simulink model "ofdm_txrx_alamouti"  (Simulink 6.3)
settings: Solver=ode45  StartTime=0.0  StopTime=45000  RelTol=1e-3
InitFcn:
  | ofdm_rx_alamouti_init
StartFcn:
  | ofdm_rx_alamouti_init
values from neighbouring files (symbols this model uses):
  CPLength = 16    [ofdm_tx_alamouti_init.m]
  CRCPolynomial = hex2dec('04c11db7')    [calcTxCRC.m]    (also in ofdm_tx_alamouti_init.m)
  hi = zeros(1,length(cci))    [ofdm_rx_alamouti_init.m]
  hr = zeros(1,length(ccr))    [ofdm_rx_alamouti_init.m]
  max_CP_length = 16    [ofdm_tx_alamouti_init.m]
  max_num_baseRateSymbols = 31    [ofdm_tx_alamouti_init.m]
  max_num_subcarriers = 64    [ofdm_tx_alamouti_init.m]
  max_num_trainingSymbols = 15    [ofdm_tx_alamouti_init.m]
  max_OFDM_symbols = 2047    [ofdm_tx_alamouti_init.m]
  modConstellation_qam16 = 0.75*[1 3 -1 -3]./3    [ofdm_tx_alamouti_init.m]
  modConstellation_qam256 = 0.9375*[3 1 5 7 11 9 13 15 -3 -1 -5 -7 -11 -9 -13 -15]./15    [ofdm_tx_alamouti_init.m]
  modConstellation_qam64 = 0.875*[3 1 5 7 -3 -1 -5 -7]./7    [ofdm_tx_alamouti_init.m]
  modConstellation_qpsk = [1 -1]./sqrt(2)    [ofdm_tx_alamouti_init.m]
  numBaseRateSymbols = 2    [ofdm_tx_alamouti_init.m]
  numFullRateSymbols = numFullRateSymbols + mod(numFullRateSymbols, 2)    [ofdm_tx_alamouti_init.m]
  numSubcarriers = 64    [ofdm_tx_alamouti_init.m]
  numTrainingSymbols = 4    [ofdm_tx_alamouti_init.m]
  pkt_crossCorr_thresh = 0.7    [ofdm_rx_alamouti_init.m]
  pkt_energy_thresh = 0    [ofdm_rx_alamouti_init.m]
  pkt_numPayloadBytes = 24+4+20+20+500    (= 568)    [ofdm_tx_alamouti_init.m]
  pktTiming_count_payloadStart = pktTiming_count_lsStart + pktTiming_ls_num*pktTiming_ls_length    [ofdm_rx_init_packetTimingControl.m]
  preamble = 6*[0 shortsyms_10 longsyms_2]    [ofdm_tx_alamouti_init.m]
  preamble_I = real(preamble)    [ofdm_tx_alamouti_init.m]
  preamble_Q = imag(preamble)    [ofdm_tx_alamouti_init.m]
  RAM_init_size = 4096    [ofdm_tx_alamouti_init.m]
  rx_longCorrCountLoad = 251-16    (= 235)    [ofdm_rx_alamouti_init.m]
  rx_longCorrThresh = 3000    [ofdm_rx_alamouti_init.m]
  RxDataScrambling_Seq = [40 198 78 63 82 173 102 245 48 111 172 115 147 230 216 93 72 65 62 2 205 242 122 90 128 83 105 97 73 10 5 252]    [ofdm_tx_alamouti_init.m]
  simOnly_numSamples = length(preamble)+( (numSubcarriers+CPLength)*(numTrainingSymbols + numBaseRateSymbols + numFullRateSymbols) )    [ofdm_tx_alamouti_init.m]
  train = [train train]    [ofdm_tx_alamouti_init.m]
  TxDataScrambling_Seq = [40 198 78 63 82 173 102 245 48 111 172 115 147 230 216 93 72 65 62 2 205 242 122 90 128 83 105 97 73 10 5 252]    [ofdm_tx_alamouti_init.m]
undefined symbols (not defined in the file or in its folder): CRC_table_gen, gcbh, interpFilter_4, xlBlockLoadCallback
libraries: xbsIndex_r4 x4373, dspmlti4 x4, dspsigops x4, commchan3 x2, simulink x2, commrflib2 x2, commsink2 x2, dspsrcs4 x1
(part 2 of 39)

== system ofdm_txrx_alamouti/BRAM Interface/PLB Pkt Buffer ==
  BRAM_Dout : Inport
  WEn : Inport  Port=2
  Addr : Inport  Port=3
  AddrIn : xbsIndex_r4/Gateway In  arith_type=Unsigned  n_bits=32  bin_pt=0  quantization="Round  (unbiased: +/- Inf)"  overflow=Saturate  period=1  dbl_ovrd=off  timing_constraint=None  locs_specified=off  LOCs={}  block_type=gatewayin
      settings: infoedit="Gateway in block.  Converts inputs of type Simulink integer, double and fixed point to  Xilinx fixed point type.<P><P>Hardware notes:  In hardware these blocks become top level input ports."  block_version=8.2.02
  Byte0 : xbsIndex_r4/Slice  nbits=8  boolean_output=off  mode=Lower Bit Location + Width  bit1=0  base1=MSB of Input  bit0=0  base0=LSB of Input  dbl_ovrd=off  block_type=slice
      settings: infoedit="Extracts a given range of bits from each input sample and presents it at the output.  The output type is ordinarily unsigned with binary point at zero, but can be Boolean when the slice is one bit wide.<P><P>Hardware notes: In hardware this block costs nothing."  block_version=8.2.02
  Byte1 : xbsIndex_r4/Slice  nbits=8  boolean_output=off  mode=Lower Bit Location + Width  bit1=0  base1=MSB of Input  bit0=8  base0=LSB of Input  dbl_ovrd=off  block_type=slice
      settings: infoedit="Extracts a given range of bits from each input sample and presents it at the output.  The output type is ordinarily unsigned with binary point at zero, but can be Boolean when the slice is one bit wide.<P><P>Hardware notes: In hardware this block costs nothing."  block_version=8.2.02
  Byte2 : xbsIndex_r4/Slice  nbits=8  boolean_output=off  mode=Lower Bit Location + Width  bit1=0  base1=MSB of Input  bit0=16  base0=LSB of Input  dbl_ovrd=off  block_type=slice
      settings: infoedit="Extracts a given range of bits from each input sample and presents it at the output.  The output type is ordinarily unsigned with binary point at zero, but can be Boolean when the slice is one bit wide.<P><P>Hardware notes: In hardware this block costs nothing."  block_version=8.2.02
  Byte3 : xbsIndex_r4/Slice  nbits=8  boolean_output=off  mode=Lower Bit Location + Width  bit1=0  base1=MSB of Input  bit0=24  base0=LSB of Input  dbl_ovrd=off  block_type=slice
      settings: infoedit="Extracts a given range of bits from each input sample and presents it at the output.  The output type is ordinarily unsigned with binary point at zero, but can be Boolean when the slice is one bit wide.<P><P>Hardware notes: In hardware this block costs nothing."  block_version=8.2.02
  Byte4 : xbsIndex_r4/Slice  nbits=8  boolean_output=off  mode=Lower Bit Location + Width  bit1=0  base1=MSB of Input  bit0=32  base0=LSB of Input  dbl_ovrd=off  block_type=slice
      settings: infoedit="Extracts a given range of bits from each input sample and presents it at the output.  The output type is ordinarily unsigned with binary point at zero, but can be Boolean when the slice is one bit wide.<P><P>Hardware notes: In hardware this block costs nothing."  block_version=8.2.02
  Byte5 : xbsIndex_r4/Slice  nbits=8  boolean_output=off  mode=Lower Bit Location + Width  bit1=0  base1=MSB of Input  bit0=40  base0=LSB of Input  dbl_ovrd=off  block_type=slice
      settings: infoedit="Extracts a given range of bits from each input sample and presents it at the output.  The output type is ordinarily unsigned with binary point at zero, but can be Boolean when the slice is one bit wide.<P><P>Hardware notes: In hardware this block costs nothing."  block_version=8.2.02
  Byte6 : xbsIndex_r4/Slice  nbits=8  boolean_output=off  mode=Lower Bit Location + Width  bit1=0  base1=MSB of Input  bit0=48  base0=LSB of Input  dbl_ovrd=off  block_type=slice
      settings: infoedit="Extracts a given range of bits from each input sample and presents it at the output.  The output type is ordinarily unsigned with binary point at zero, but can be Boolean when the slice is one bit wide.<P><P>Hardware notes: In hardware this block costs nothing."  block_version=8.2.02
  Byte7 : xbsIndex_r4/Slice  nbits=8  boolean_output=off  mode=Lower Bit Location + Width  bit1=0  base1=MSB of Input  bit0=56  base0=LSB of Input  dbl_ovrd=off  block_type=slice
      settings: infoedit="Extracts a given range of bits from each input sample and presents it at the output.  The output type is ordinarily unsigned with binary point at zero, but can be Boolean when the slice is one bit wide.<P><P>Hardware notes: In hardware this block costs nothing."  block_version=8.2.02
  Byte8 : xbsIndex_r4/Slice  nbits=9  boolean_output=off  mode=Lower Bit Location + Width  bit1=0  base1=MSB of Input  bit0=3  base0=LSB of Input  dbl_ovrd=off  block_type=slice
      settings: infoedit="Extracts a given range of bits from each input sample and presents it at the output.  The output type is ordinarily unsigned with binary point at zero, but can be Boolean when the slice is one bit wide.<P><P>Hardware notes: In hardware this block costs nothing."  block_version=8.2.02
  Concat : xbsIndex_r4/Concat  num_inputs=8  dbl_ovrd=off  block_type=concat
      settings: block_version=8.2.02
  Constant : xbsIndex_r4/Constant  arith_type=Unsigned  const=0  n_bits=1  bin_pt=0  explicit_period=off  period=1  equ=P=C  opselect=C  inp2=PCIN>>17  opr=+  inp1=P  carry=CIN  dbl_ovrd=off  block_type=constant
      settings: block_version=8.2.02
  DataIn : xbsIndex_r4/Gateway In  arith_type=Unsigned  n_bits=64  bin_pt=0  quantization="Round  (unbiased: +/- Inf)"  overflow=Saturate  period=1  dbl_ovrd=off  timing_constraint=None  locs_specified=off  LOCs={}  block_type=gatewayin
      settings: infoedit="Gateway in block.  Converts inputs of type Simulink integer, double and fixed point to  Xilinx fixed point type.<P><P>Hardware notes:  In hardware these blocks become top level input ports."  block_version=8.2.02
  DataOut : xbsIndex_r4/Gateway Out  hdl_port=on  timing_constraint=None  locs_specified=off  LOCs={}  block_type=gatewayout
      settings: infoedit="Gateway out block.  Converts Xilinx fixed point inputs into ouputs of type Simulink integer, double, or fixed point.<P><P>Hardware notes:  In hardware these blocks become top level output ports or are discarded, depending on how they are configured."  block_version=8.2.02
  Delay : xbsIndex_r4/Delay  en=on  latency=1  dbl_ovrd=off  reg_retiming=off  block_type=delay
      settings: infoedit=Hardware notes: A delay line is a chain, each link of which is an SRL16 followed by a flip-flop. If register retiming is enabled, the delay line is a chain of flip-flops.  block_version=8.2.02
  Disregard Subsystem : xbsIndex_r4/Disregard Subsystem  Tag=discardX  block_type=disregard
      settings: block_version=8.2.02
  Gateway Out{,1..3} (4 alike) : xbsIndex_r4/Gateway Out  hdl_port=off  timing_constraint=None  locs_specified=off  LOCs={}  block_type=gatewayout
      settings: infoedit="Gateway out block.  Converts Xilinx fixed point inputs into ouputs of type Simulink integer, double, or fixed point.<P><P>Hardware notes:  In hardware these blocks become top level output ports or are discarded, depending on how they are configured."  block_version=8.2.02
  ROM : xbsIndex_r4/ROM  depth=RAM_init_size/8  initVector=RAM_init_values(8:8:end)  distributed_mem=Block RAM  rst=off  init_reg=0  en=off  latency=1  arith_type=Unsigned  n_bits=8  bin_pt=0  dbl_ovrd=off  optimize=Area  use_rpm=on  block_type=sprom
      settings: block_version=8.2.02
  ROM1 : xbsIndex_r4/ROM  depth=RAM_init_size/8  initVector=RAM_init_values(7:8:end)  distributed_mem=Block RAM  rst=off  init_reg=0  en=off  latency=1  arith_type=Unsigned  n_bits=8  bin_pt=0  dbl_ovrd=off  optimize=Area  use_rpm=on  block_type=sprom
      settings: block_version=8.2.02
  ROM2 : xbsIndex_r4/ROM  depth=RAM_init_size/8  initVector=RAM_init_values(6:8:end)  distributed_mem=Block RAM  rst=off  init_reg=0  en=off  latency=1  arith_type=Unsigned  n_bits=8  bin_pt=0  dbl_ovrd=off  optimize=Area  use_rpm=on  block_type=sprom
      settings: block_version=8.2.02
  ROM3 : xbsIndex_r4/ROM  depth=RAM_init_size/8  initVector=RAM_init_values(5:8:end)  distributed_mem=Block RAM  rst=off  init_reg=0  en=off  latency=1  arith_type=Unsigned  n_bits=8  bin_pt=0  dbl_ovrd=off  optimize=Area  use_rpm=on  block_type=sprom
      settings: block_version=8.2.02
  ROM4 : xbsIndex_r4/ROM  depth=RAM_init_size/8  initVector=RAM_init_values(4:8:end)  distributed_mem=Block RAM  rst=off  init_reg=0  en=off  latency=1  arith_type=Unsigned  n_bits=8  bin_pt=0  dbl_ovrd=off  optimize=Area  use_rpm=on  block_type=sprom
      settings: block_version=8.2.02
  ROM5 : xbsIndex_r4/ROM  depth=RAM_init_size/8  initVector=RAM_init_values(3:8:end)  distributed_mem=Block RAM  rst=off  init_reg=0  en=off  latency=1  arith_type=Unsigned  n_bits=8  bin_pt=0  dbl_ovrd=off  optimize=Area  use_rpm=on  block_type=sprom
      settings: block_version=8.2.02
  ROM6 : xbsIndex_r4/ROM  depth=RAM_init_size/8  initVector=RAM_init_values(2:8:end)  distributed_mem=Block RAM  rst=off  init_reg=0  en=off  latency=1  arith_type=Unsigned  n_bits=8  bin_pt=0  dbl_ovrd=off  optimize=Area  use_rpm=on  block_type=sprom
      settings: block_version=8.2.02
  ROM7 : xbsIndex_r4/ROM  depth=RAM_init_size/8  initVector=RAM_init_values(1:8:end)  distributed_mem=Block RAM  rst=off  init_reg=0  en=off  latency=1  arith_type=Unsigned  n_bits=8  bin_pt=0  dbl_ovrd=off  optimize=Area  use_rpm=on  block_type=sprom
      settings: block_version=8.2.02
  Relational : xbsIndex_r4/Relational  mode=a!=b  en=off  latency=0  dbl_ovrd=off  block_type=relational
      settings: block_version=8.2.02
  Scope : Scope  NumInputPorts=4
      settings: YMin=0~0~0~0  YMax=15~1e+019~2e+019~1  SaveName=ScopeData52
  Single Port RAM10 : xbsIndex_r4/Single Port RAM  depth=RAM_init_size/8  initVector=RAM_init_values(3:8:end)  distributed_mem=Block RAM  write_mode=Read After Write  rst=off  init_reg=0  en=off  latency=1  dbl_ovrd=off  optimize=Area  use_rpm=on  block_type=spram
      settings: block_version=8.2.02
  Single Port RAM11 : xbsIndex_r4/Single Port RAM  depth=RAM_init_size/8  initVector=RAM_init_values(4:8:end)  distributed_mem=Block RAM  write_mode=Read After Write  rst=off  init_reg=0  en=off  latency=1  dbl_ovrd=off  optimize=Area  use_rpm=on  block_type=spram
      settings: block_version=8.2.02
  Single Port RAM12 : xbsIndex_r4/Single Port RAM  depth=RAM_init_size/8  initVector=RAM_init_values(5:8:end)  distributed_mem=Block RAM  write_mode=Read After Write  rst=off  init_reg=0  en=off  latency=1  dbl_ovrd=off  optimize=Area  use_rpm=on  block_type=spram
      settings: block_version=8.2.02
  Single Port RAM13 : xbsIndex_r4/Single Port RAM  depth=RAM_init_size/8  initVector=RAM_init_values(6:8:end)  distributed_mem=Block RAM  write_mode=Read After Write  rst=off  init_reg=0  en=off  latency=1  dbl_ovrd=off  optimize=Area  use_rpm=on  block_type=spram
      settings: block_version=8.2.02
  Single Port RAM14 : xbsIndex_r4/Single Port RAM  depth=RAM_init_size/8  initVector=RAM_init_values(7:8:end)  distributed_mem=Block RAM  write_mode=Read After Write  rst=off  init_reg=0  en=off  latency=1  dbl_ovrd=off  optimize=Area  use_rpm=on  block_type=spram
      settings: block_version=8.2.02
  Single Port RAM15 : xbsIndex_r4/Single Port RAM  depth=RAM_init_size/8  initVector=RAM_init_values(8:8:end)  distributed_mem=Block RAM  write_mode=Read After Write  rst=off  init_reg=0  en=off  latency=1  dbl_ovrd=off  optimize=Area  use_rpm=on  block_type=spram
      settings: block_version=8.2.02
  Single Port RAM8 : xbsIndex_r4/Single Port RAM  depth=RAM_init_size/8  initVector=RAM_init_values(1:8:end)  distributed_mem=Block RAM  write_mode=Read After Write  rst=off  init_reg=0  en=off  latency=1  dbl_ovrd=off  optimize=Area  use_rpm=on  block_type=spram
      settings: block_version=8.2.02
  Single Port RAM9 : xbsIndex_r4/Single Port RAM  depth=RAM_init_size/8  initVector=RAM_init_values(2:8:end)  distributed_mem=Block RAM  write_mode=Read After Write  rst=off  init_reg=0  en=off  latency=1  dbl_ovrd=off  optimize=Area  use_rpm=on  block_type=spram
      settings: block_version=8.2.02
  WEn0 : xbsIndex_r4/Slice  nbits=1  boolean_output=on  mode=Lower Bit Location + Width  bit1=0  base1=MSB of Input  bit0=0  base0=LSB of Input  dbl_ovrd=off  block_type=slice
      settings: infoedit="Extracts a given range of bits from each input sample and presents it at the output.  The output type is ordinarily unsigned with binary point at zero, but can be Boolean when the slice is one bit wide.<P><P>Hardware notes: In hardware this block costs nothing."  block_version=8.2.02
  WEn1 : xbsIndex_r4/Slice  nbits=1  boolean_output=on  mode=Lower Bit Location + Width  bit1=0  base1=MSB of Input  bit0=1  base0=LSB of Input  dbl_ovrd=off  block_type=slice
      settings: infoedit="Extracts a given range of bits from each input sample and presents it at the output.  The output type is ordinarily unsigned with binary point at zero, but can be Boolean when the slice is one bit wide.<P><P>Hardware notes: In hardware this block costs nothing."  block_version=8.2.02
  WEn2 : xbsIndex_r4/Slice  nbits=1  boolean_output=on  mode=Lower Bit Location + Width  bit1=0  base1=MSB of Input  bit0=2  base0=LSB of Input  dbl_ovrd=off  block_type=slice
      settings: infoedit="Extracts a given range of bits from each input sample and presents it at the output.  The output type is ordinarily unsigned with binary point at zero, but can be Boolean when the slice is one bit wide.<P><P>Hardware notes: In hardware this block costs nothing."  block_version=8.2.02
  WEn3 : xbsIndex_r4/Slice  nbits=1  boolean_output=on  mode=Lower Bit Location + Width  bit1=0  base1=MSB of Input  bit0=3  base0=LSB of Input  dbl_ovrd=off  block_type=slice
      settings: infoedit="Extracts a given range of bits from each input sample and presents it at the output.  The output type is ordinarily unsigned with binary point at zero, but can be Boolean when the slice is one bit wide.<P><P>Hardware notes: In hardware this block costs nothing."  block_version=8.2.02
  WEn4 : xbsIndex_r4/Slice  nbits=1  boolean_output=on  mode=Lower Bit Location + Width  bit1=0  base1=MSB of Input  bit0=4  base0=LSB of Input  dbl_ovrd=off  block_type=slice
      settings: infoedit="Extracts a given range of bits from each input sample and presents it at the output.  The output type is ordinarily unsigned with binary point at zero, but can be Boolean when the slice is one bit wide.<P><P>Hardware notes: In hardware this block costs nothing."  block_version=8.2.02
  WEn5 : xbsIndex_r4/Slice  nbits=1  boolean_output=on  mode=Lower Bit Location + Width  bit1=0  base1=MSB of Input  bit0=5  base0=LSB of Input  dbl_ovrd=off  block_type=slice
      settings: infoedit="Extracts a given range of bits from each input sample and presents it at the output.  The output type is ordinarily unsigned with binary point at zero, but can be Boolean when the slice is one bit wide.<P><P>Hardware notes: In hardware this block costs nothing."  block_version=8.2.02
  WEn6 : xbsIndex_r4/Slice  nbits=1  boolean_output=on  mode=Lower Bit Location + Width  bit1=0  base1=MSB of Input  bit0=6  base0=LSB of Input  dbl_ovrd=off  block_type=slice
      settings: infoedit="Extracts a given range of bits from each input sample and presents it at the output.  The output type is ordinarily unsigned with binary point at zero, but can be Boolean when the slice is one bit wide.<P><P>Hardware notes: In hardware this block costs nothing."  block_version=8.2.02
  WEn7 : xbsIndex_r4/Slice  nbits=1  boolean_output=on  mode=Lower Bit Location + Width  bit1=0  base1=MSB of Input  bit0=7  base0=LSB of Input  dbl_ovrd=off  block_type=slice
      settings: infoedit="Extracts a given range of bits from each input sample and presents it at the output.  The output type is ordinarily unsigned with binary point at zero, but can be Boolean when the slice is one bit wide.<P><P>Hardware notes: In hardware this block costs nothing."  block_version=8.2.02
  WEnIn : xbsIndex_r4/Gateway In  arith_type=Unsigned  n_bits=8  bin_pt=0  quantization="Round  (unbiased: +/- Inf)"  overflow=Saturate  period=1  dbl_ovrd=off  timing_constraint=None  locs_specified=off  LOCs={}  block_type=gatewayin
      settings: infoedit="Gateway in block.  Converts inputs of type Simulink integer, double and fixed point to  Xilinx fixed point type.<P><P>Hardware notes:  In hardware these blocks become top level input ports."  block_version=8.2.02
  Data : Outport
  wires:
  DataIn/1 -> Byte0/1, Byte1/1, Byte2/1, Byte3/1, Byte4/1, Byte5/1, Byte6/1, Byte7/1, Delay/1
  AddrIn/1 -> Byte8/1
  ROM/1 -> Concat/1
  ROM1/1 -> Concat/2
  ROM2/1 -> Concat/3
  ROM3/1 -> Concat/4
  ROM4/1 -> Concat/5
  ROM5/1 -> Concat/6
  ROM6/1 -> Concat/7
  ROM7/1 -> Concat/8
  Byte7/1 -> Single Port RAM15/2
  Byte6/1 -> Single Port RAM14/2
  Byte5/1 -> Single Port RAM13/2
  Byte4/1 -> Single Port RAM12/2
  Byte3/1 -> Single Port RAM11/2
  Byte2/1 -> Single Port RAM10/2
  Byte1/1 -> Single Port RAM9/2
  Byte0/1 -> Single Port RAM8/2
  WEn0/1 -> Single Port RAM8/3
  WEn1/1 -> Single Port RAM9/3
  WEn2/1 -> Single Port RAM10/3
  WEn3/1 -> Single Port RAM11/3
  WEn4/1 -> Single Port RAM12/3
  WEn5/1 -> Single Port RAM13/3
  WEn6/1 -> Single Port RAM14/3
  WEn7/1 -> Single Port RAM15/3
  WEnIn/1 -> WEn6/1, WEn7/1, WEn5/1, WEn4/1, WEn3/1, WEn2/1, WEn1/1, WEn0/1, Relational/1
  WEn/1 -> WEnIn/1
  BRAM_Dout/1 -> DataIn/1
  Addr/1 -> AddrIn/1
  Concat/1 -> DataOut/1, Gateway Out1/1
  DataOut/1 -> Data/1
  [BRAM_Rx_WrAddr] -> Single Port RAM9/1, Single Port RAM8/1, Single Port RAM10/1, Single Port RAM11/1, Single Port RAM12/1, Single Port RAM13/1, Single Port RAM14/1, Single Port RAM15/1
  Gateway Out/1 -> Scope/1
  Gateway Out1/1 -> Scope/2
  Gateway Out2/1 -> Scope/3
  Gateway Out3/1 -> Scope/4
  Constant/1 -> Relational/2
  Relational/1 -> Delay/2, Gateway Out3/1
  Delay/1 -> Gateway Out2/1
  [BRAM_Tx_RdAddr] -> ROM6/1, ROM7/1, Gateway Out/1, ROM5/1, ROM4/1, ROM3/1, ROM2/1, ROM1/1, ROM/1

== system ofdm_txrx_alamouti/OFDM Rx Alamouti/ADCs & Ant Selection ==
  "A I " : Inport
  "A Q " : Inport  Port=2
  "B I " : Inport  Port=3
  "B Q " : Inport  Port=4
  PktDetReset : Inport  Port=5
  Antenna Selection : Subsystem
  Down Sample{,1..3} (4 alike) : xbsIndex_r4/Down Sample  sample_ratio=4  sample_phase="Last Value of Frame  (most efficient)"  en=off  latency=1  dbl_ovrd=off  block_type=dsamp
      settings: block_version=8.2.02
  Rx_AntA_ADCI_DV : xbsIndex_r4/Gateway In  arith_type="Signed  (2's comp)"  n_bits=14  bin_pt=13  quantization="Round  (unbiased: +/- Inf)"  overflow=Saturate  period=1  dbl_ovrd=off  timing_constraint=None  locs_specified=off  LOCs={}  block_type=gatewayin
      settings: infoedit="Gateway in block.  Converts inputs of type Simulink integer, double and fixed point to  Xilinx fixed point type.<P><P>Hardware notes:  In hardware these blocks become top level input ports."  block_version=VER_STRING_GOES_HERE
  Rx_AntA_ADCQ_DV : xbsIndex_r4/Gateway In  arith_type="Signed  (2's comp)"  n_bits=14  bin_pt=13  quantization="Round  (unbiased: +/- Inf)"  overflow=Saturate  period=1  dbl_ovrd=off  timing_constraint=None  locs_specified=off  LOCs={}  block_type=gatewayin
      settings: infoedit="Gateway in block.  Converts inputs of type Simulink integer, double and fixed point to  Xilinx fixed point type.<P><P>Hardware notes:  In hardware these blocks become top level input ports."  block_version=VER_STRING_GOES_HERE
  Rx_AntB_ADCI_DV : xbsIndex_r4/Gateway In  arith_type="Signed  (2's comp)"  n_bits=14  bin_pt=13  quantization="Round  (unbiased: +/- Inf)"  overflow=Saturate  period=1  dbl_ovrd=off  timing_constraint=None  locs_specified=off  LOCs={}  block_type=gatewayin
      settings: infoedit="Gateway in block.  Converts inputs of type Simulink integer, double and fixed point to  Xilinx fixed point type.<P><P>Hardware notes:  In hardware these blocks become top level input ports."  block_version=VER_STRING_GOES_HERE
  Rx_AntB_ADCQ_DV : xbsIndex_r4/Gateway In  arith_type="Signed  (2's comp)"  n_bits=14  bin_pt=13  quantization="Round  (unbiased: +/- Inf)"  overflow=Saturate  period=1  dbl_ovrd=off  timing_constraint=None  locs_specified=off  LOCs={}  block_type=gatewayin
      settings: infoedit="Gateway in block.  Converts inputs of type Simulink integer, double and fixed point to  Xilinx fixed point type.<P><P>Hardware notes:  In hardware these blocks become top level input ports."  block_version=VER_STRING_GOES_HERE
  I : Outport
  Q : Outport  Port=2
  tag scope: AntA_ADC_I_Filt=global, AntA_ADC_Q_Filt=global
  wires:
  "B Q "/1 -> Rx_AntB_ADCQ_DV/1
  "B I "/1 -> Rx_AntB_ADCI_DV/1
  "A Q "/1 -> Rx_AntA_ADCQ_DV/1
  "A I "/1 -> Rx_AntA_ADCI_DV/1
  Rx_AntA_ADCI_DV/1 -> Down Sample/1
  Rx_AntB_ADCQ_DV/1 -> Down Sample3/1
  Rx_AntB_ADCI_DV/1 -> Down Sample2/1
  Rx_AntA_ADCQ_DV/1 -> Down Sample1/1
  Down Sample/1 -> Antenna Selection/1(AntA_I)
  Down Sample1/1 -> Antenna Selection/2(AntA_Q)
  Down Sample2/1 -> Antenna Selection/3(AntB_I)
  Down Sample3/1 -> Antenna Selection/4(AntB_Q)
  Antenna Selection/1(I) -> I/1, [AntA_ADC_I_Filt]
  Antenna Selection/2(Q) -> Q/1, [AntA_ADC_Q_Filt]
  PktDetReset/1 -> Antenna Selection/5(PktDetReset)

== system ofdm_txrx_alamouti/OFDM Rx Alamouti/Coarse Freq Correction1 ==
  PktDet : Inport
  Reset : Inport  Port=2
  AntA I : Inport  Port=3
  AntA Q : Inport  Port=4
  Fine Freq : Inport  Port=5
  PktDone : Inport  Port=6
  A*B : Subsystem
  Add1 : xbsIndex_r4/AddSub  mode=Addition  use_carryin=off  use_carryout=off  en=off  latency=0  precision=User Defined  arith_type=Unsigned  n_bits=32  bin_pt=32  quantization=Truncate  overflow=Wrap  dbl_ovrd=off  use_behavioral_HDL=on  pipelined=off  use_rpm=on  block_type=addsub
      settings: xl_area=[9 0 0 16 0 0 0]  block_version=VER_STRING_GOES_HERE
  Bus Creator1 : BusCreator  Inputs=2
  Coarse Freq Offset Calc Combo : Subsystem
  Complex to Magnitude-Angle : ComplexToMagnitudeAngle
  Complex to Real-Imag : ComplexToRealImag
  Convert : xbsIndex_r4/Convert  arith_type="Signed  (2's comp)"  n_bits=14  bin_pt=13  quantization=Truncate  overflow=Flag as error  latency=0  dbl_ovrd=off  pipeline=off  block_type=convert
      settings: xl_area=[0 0 0 0 0 0 0]  block_version=VER_STRING_GOES_HERE
  Convert1 : xbsIndex_r4/Convert  arith_type="Signed  (2's comp)"  n_bits=14  bin_pt=13  quantization=Truncate  overflow=Flag as error  latency=0  dbl_ovrd=off  pipeline=off  block_type=convert
      settings: xl_area=[0 0 0 0 0 0 0]  block_version=VER_STRING_GOES_HERE
  DDS : Subsystem
  Down Sample : xbsIndex_r4/Down Sample  sample_ratio=2  sample_phase="Last Value of Frame  (most efficient)"  en=off  latency=1  dbl_ovrd=off  block_type=dsamp
      settings: block_version=VER_STRING_GOES_HERE
  Down Sample1 : xbsIndex_r4/Down Sample  sample_ratio=2  sample_phase="Last Value of Frame  (most efficient)"  en=off  latency=1  dbl_ovrd=off  block_type=dsamp
      settings: block_version=VER_STRING_GOES_HERE
  Freq Correction Sinusoids : Scope  NumInputPorts=5
      settings: TimeRange=5000  YMin=0~0~-1~-1~0  YMax=0.0004~2.5e-005~1~1~1  SaveName=ScopeData11
  Gateway Out{,1..3} (4 alike) : xbsIndex_r4/Gateway Out  hdl_port=off  timing_constraint=None  locs_specified=off  LOCs={}  block_type=gatewayout
      settings: infoedit="Gateway out block.  Converts Xilinx fixed point inputs into ouputs of type Simulink integer, double, or fixed point.<P><P>Hardware notes:  In hardware these blocks become top level output ports or are discarded, depending on how they are configured."  xl_area=[0 0 0 0 0 0 0]  block_version=VER_STRING_GOES_HERE
  Mux2 : Mux  Inputs=2
  Product : Product  RndMeth=Zero
  Real-Imag to Complex : RealImagToComplex
  Register : xbsIndex_r4/Register  init=0  rst=off  en=on  dbl_ovrd=off  block_type=register
      settings: block_version=8.2.02
  To Workspace : ToWorkspace  VariableName=AntA_fromSim  SampleTime=-1  SaveFormat=Array
  Up Sample : xbsIndex_r4/Up Sample  sample_ratio=2  copy_samples=on  dbl_ovrd=off  block_type=usamp
      settings: infoedit="Up samples input data.  Inserted values  can be zeros or copies of the most recent input sample.<P><P>Hardware notes: No hardware is needed if inserted values are copies of the input sample; otherwise, a mux and single bit flip-flop are used."  block_version=8.2.01
  " AntA I" : Outport
  " AntA Q" : Outport  Port=2
  tag scope: CFO_PhaseInc=global, Rx_CFO_PhaseInc=global
  wires:
  Up Sample/1 -> Register/1
  Down Sample1/1 -> [Rx_CFO_PhaseInc]
  PktDone/1 -> Register/2
  Register/1 -> Down Sample1/1
  PktDet/1 -> Coarse Freq Offset Calc Combo/4(PktDet)
  Coarse Freq Offset Calc Combo/1(Phase Inc) -> Add1/1
  Reset/1 -> DDS/2(Rst), Coarse Freq Offset Calc Combo/3(Reset)
  Add1/1 -> Up Sample/1, DDS/1(PhaseInc), Gateway Out3/1, [CFO_PhaseInc]
  Fine Freq/1 -> Down Sample/1
  Down Sample/1 -> Add1/2, Gateway Out2/1
  Complex to Magnitude-Angle/2 -> Freq Correction Sinusoids/5
  Product/1 -> Complex to Magnitude-Angle/1
  Real-Imag to Complex/1 -> Product/1
  Convert1/1 -> " AntA Q"/1, Coarse Freq Offset Calc Combo/2(Q In)
  Convert/1 -> " AntA I"/1, Coarse Freq Offset Calc Combo/1(I In), To Workspace/1
  AntA Q/1 -> A*B/2(Im[A])
  AntA I/1 -> A*B/1(Re[A])
  Gateway Out3/1 -> Freq Correction Sinusoids/1
  Gateway Out2/1 -> Freq Correction Sinusoids/2
  Complex to Real-Imag/2 -> Bus Creator1/2
  Complex to Real-Imag/1 -> Bus Creator1/1
  [freqOffset] -> Product/2, Complex to Real-Imag/1
  Bus Creator1/1 -> Freq Correction Sinusoids/4
  Mux2/1 -> Freq Correction Sinusoids/3
  Gateway Out/1 -> Real-Imag to Complex/2, Mux2/2
  Gateway Out1/1 -> Real-Imag to Complex/1, Mux2/1
  DDS/2(I) -> Gateway Out1/1, A*B/4(Re[B])
  DDS/1(Q) -> Gateway Out/1, A*B/3(Im[B])
  A*B/2(Im1) -> Convert1/1
  A*B/1(Re) -> Convert/1

== system ofdm_txrx_alamouti/OFDM Rx Alamouti/Equalizer & Packetizer ==
  Payload : Inport
  Y_I : Inport  Port=2
  Y_Q : Inport  Port=3
  H-I : Inport  Port=4
  H-Q : Inport  Port=5
  SubCar Index : Inport  Port=6
  Sym Index : Inport  Port=7
  Vin : Inport  Port=8
  AddSub : xbsIndex_r4/AddSub  mode=Addition  use_carryin=off  use_carryout=off  en=off  latency=0  precision=User Defined  arith_type="Signed  (2's comp)"  n_bits=32  bin_pt=28  quantization=Truncate  overflow=Wrap  dbl_ovrd=off  use_behavioral_HDL=off  pipelined=off  use_rpm=on  block_type=addsub
      settings: block_version=VER_STRING_GOES_HERE
  Alamouti Combining : Subsystem
  Convert{1..2,4..5} (4 alike) : xbsIndex_r4/Convert  arith_type="Signed  (2's comp)"  n_bits=14  bin_pt=13  quantization=Truncate  overflow=Wrap  latency=0  dbl_ovrd=off  pipeline=off  block_type=convert
      settings: xl_area=[0, 0, 0, 0, 0, 0, 0]  block_version=VER_STRING_GOES_HERE
  Loop Filter A : Subsystem
  Loop Filter B : Subsystem
  Packet_Constructor : Subsystem
  Phase Adjust : Subsystem
  Phase Noise Tracker : Subsystem
  Pilot_Extractor : Subsystem
  Pkt Constructor Inputs : Scope  NumInputPorts=6
      settings: TimeRange=8000  YMin=-0.75~-0.4~0~0~0~0  YMax=0.75~0.4~70~1~15~1  SaveName=ScopeData29
  Post-PhaseAdj : commsink2/Discrete-Time Scatter Plot Scope  Tag=ScatterPlot  sampPerSymb=1  offsetEye=0  numTraces=1500  numNewFrames=10  LineMarkers=.  LineColors=b  fading=on  render=on  AxisGrid=on  xMin=-1.09999847412109  xMax=1.10496788024902  yMin=-1.09999847412109  yMax=1.10496788024902  inphaseLabel=In-phase Amplitude  quadratureLabel=Quadrature Amplitude  openScopeAtSimStart=off  FrameNumber=off  FigPos=[360 362 560 560]  figTitle=Scatter Plot  numLinesMax=8  block_type_=scatter
  Real-Imag to Complex1 : RealImagToComplex
  Rx_debug_EQ_I : xbsIndex_r4/Gateway Out  hdl_port=on  timing_constraint=None  locs_specified=off  LOCs={}  block_type=gatewayout
      settings: infoedit="Gateway out block.  Converts Xilinx fixed point inputs into ouputs of type Simulink integer, double, or fixed point.<P><P>Hardware notes:  In hardware these blocks become top level output ports or are discarded, depending on how they are configured."  xl_area=[0 0 0 0 1 0 0]  block_version=VER_STRING_GOES_HERE
  Rx_debug_EQ_Q : xbsIndex_r4/Gateway Out  hdl_port=on  timing_constraint=None  locs_specified=off  LOCs={}  block_type=gatewayout
      settings: infoedit="Gateway out block.  Converts Xilinx fixed point inputs into ouputs of type Simulink integer, double, or fixed point.<P><P>Hardware notes:  In hardware these blocks become top level output ports or are discarded, depending on how they are configured."  xl_area=[0 0 0 0 1 0 0]  block_version=VER_STRING_GOES_HERE
  Rx_debug_PhaseCorrect : xbsIndex_r4/Gateway Out  hdl_port=on  timing_constraint=None  locs_specified=off  LOCs={}  block_type=gatewayout
      settings: infoedit="Gateway out block.  Converts Xilinx fixed point inputs into ouputs of type Simulink integer, double, or fixed point.<P><P>Hardware notes:  In hardware these blocks become top level output ports or are discarded, depending on how they are configured."  xl_area=[0 0 0 0 1 0 0]  block_version=VER_STRING_GOES_HERE
  Rx_debug_PhaseErr : xbsIndex_r4/Gateway Out  hdl_port=on  timing_constraint=None  locs_specified=off  LOCs={}  block_type=gatewayout
      settings: infoedit="Gateway out block.  Converts Xilinx fixed point inputs into ouputs of type Simulink integer, double, or fixed point.<P><P>Hardware notes:  In hardware these blocks become top level output ports or are discarded, depending on how they are configured."  xl_area=[0 0 0 0 1 0 0]  block_version=VER_STRING_GOES_HERE
  Scaling : Subsystem
  Up Sample{1..4} (4 alike) : xbsIndex_r4/Up Sample  sample_ratio=2  copy_samples=on  dbl_ovrd=off  block_type=usamp
      settings: infoedit="Up samples input data.  Inserted values  can be zeros or copies of the most recent input sample.<P><P>Hardware notes: No hardware is needed if inserted values are copies of the input sample; otherwise, a mux and single bit flip-flop are used."  block_version=VER_STRING_GOES_HERE
  done{1,6..10} (6 alike) : xbsIndex_r4/Gateway Out  hdl_port=off  timing_constraint=None  locs_specified=off  LOCs={}  block_type=gatewayout
      settings: infoedit="Gateway out block.  Converts Xilinx fixed point inputs into ouputs of type Simulink integer, double, or fixed point.<P><P>Hardware notes:  In hardware these blocks become top level output ports or are discarded, depending on how they are configured."  xl_area=[0 0 0 0 1 0 0]  block_version=VER_STRING_GOES_HERE
  posedge : Subsystem
  Fine Freq : Outport
  done : Outport  Port=2
  tag scope: EQ_I=global, EQ_Q=global
  wires:
  Scaling/1(I) -> Convert4/1, done6/1, Real-Imag to Complex1/1, Packet_Constructor/1(I)
  Scaling/2(Q) -> Convert5/1, Packet_Constructor/2(Q), done7/1, Real-Imag to Complex1/2
  Scaling/3(" Xk_index") -> done1/1, Packet_Constructor/3(Xk_index)
  Scaling/4(Vout) -> done8/1, Packet_Constructor/4(Vin)
  Scaling/5(" Sym Index") -> done9/1, Packet_Constructor/5(Sym Index)
  Packet_Constructor/1(done) -> done/1, Pilot_Extractor/8(rst)
  done6/1 -> Pkt Constructor Inputs/1
  done7/1 -> Pkt Constructor Inputs/2
  done1/1 -> Pkt Constructor Inputs/3
  done8/1 -> Pkt Constructor Inputs/4
  done9/1 -> Pkt Constructor Inputs/5
  Convert4/1 -> [EQ_I], Up Sample1/1
  Convert5/1 -> [EQ_Q], Up Sample3/1
  Payload/1 -> posedge/1(D)
  posedge/1(Q) -> done10/1, Packet_Constructor/6(Start)
  done10/1 -> Pkt Constructor Inputs/6
  Pilot_Extractor/1(Phase Err) -> Loop Filter A/1(Phase Err), Phase Noise Tracker/1(Phase Err), Loop Filter B/1(Phase Err), Convert2/1
  Pilot_Extractor/2(Rst) -> Loop Filter B/2(Rst), Loop Filter A/2(Rst), Phase Noise Tracker/2(Reset)
  Pilot_Extractor/3(Vout) -> Loop Filter B/3(Vin), Loop Filter A/3(Vin), Phase Noise Tracker/3(Vin)
  Y_I/1 -> Pilot_Extractor/1(Y_I), Phase Adjust/2(Y_I)
  H-I/1 -> Pilot_Extractor/3(H-I), Phase Adjust/4(H_I)
  H-Q/1 -> Pilot_Extractor/4(H-Q), Phase Adjust/5(H_Q)
  Vin/1 -> Pilot_Extractor/7(Vin), Phase Adjust/6(Vin)
  Sym Index/1 -> Pilot_Extractor/5(Sym Index), Phase Noise Tracker/4(Sym Index), Phase Adjust/7(Sym Index)
  SubCar Index/1 -> Pilot_Extractor/6(Xk_index), Phase Adjust/8(SubCar Index)
  Y_Q/1 -> Pilot_Extractor/2(Y_Q), Phase Adjust/3(Y_Q)
  Phase Noise Tracker/1(theta) -> AddSub/2
  Loop Filter B/1(Fine Freq) -> Fine Freq/1
  Loop Filter A/1(Phase Adj) -> AddSub/1
  AddSub/1 -> Convert1/1, Phase Adjust/1(Phase)
  Convert1/1 -> Up Sample2/1
  Convert2/1 -> Up Sample4/1
  Real-Imag to Complex1/1 -> Post-PhaseAdj/1
  Phase Adjust/1(" Y_I") -> Alamouti Combining/1(Y_I)
  Phase Adjust/2(" Y_Q") -> Alamouti Combining/2(Y_Q)
  Phase Adjust/3(" H-I") -> Alamouti Combining/3(H-I)
  Phase Adjust/4(" H-Q") -> Alamouti Combining/4(H-Q)
  Phase Adjust/5(" SubCar Index") -> Alamouti Combining/5(SubCar Index)
  Phase Adjust/6(Vout) -> Alamouti Combining/6(Vin)
  Phase Adjust/7(" Sym Index") -> Alamouti Combining/7(Sym Index)
  Alamouti Combining/1(I-eq) -> Scaling/1(Eq-I)
  Alamouti Combining/2(Q-eq) -> Scaling/2(Eq-Q)
  Alamouti Combining/3(" Xk_index") -> Scaling/3(Xk_index)
  Alamouti Combining/4(Vout) -> Scaling/4(Vin)
  Alamouti Combining/5(" Sym Index") -> Scaling/5(Sym Index)
  Up Sample2/1 -> Rx_debug_PhaseCorrect/1
  Up Sample1/1 -> Rx_debug_EQ_I/1
  Up Sample3/1 -> Rx_debug_EQ_Q/1
  Up Sample4/1 -> Rx_debug_PhaseErr/1

== system ofdm_txrx_alamouti/OFDM Rx Alamouti/FFT & Chan Est ==
  Payload : Inport
  AntA I : Inport  Port=2
  AntA Q : Inport  Port=3
  Addr Gen : Subsystem
  Addr_Generator : Subsystem
  Assert : xbsIndex_r4/Assert  Orientation=left  assert_type=off  type_source=Explicitly  arith_type="Signed  (2's comp)"  n_bits=16  bin_pt=14  assert_rate=on  rate_source=Explicitly  period=2  output_port=on  block_type=assert
      settings: infoedit=Asserts a user-defined sample rate and/or type on a signal.<P><P>Hardware notes: In hardware this block costs nothing.  block_version=VER_STRING_GOES_HERE
  Channel Estimation : Subsystem
  Delay1 : xbsIndex_r4/Delay  en=off  latency=1  dbl_ovrd=off  reg_retiming=off  block_type=delay
      settings: infoedit=Hardware notes: A delay line is a chain, each link of which is an SRL16 followed by a flip-flop. If register retiming is enabled, the delay line is a chain of flip-flops.  block_version=VER_STRING_GOES_HERE
  Delay2 : xbsIndex_r4/Delay  en=off  latency=1  dbl_ovrd=off  reg_retiming=off  block_type=delay
      settings: infoedit=Hardware notes: A delay line is a chain, each link of which is an SRL16 followed by a flip-flop. If register retiming is enabled, the delay line is a chain of flip-flops.  block_version=VER_STRING_GOES_HERE
  Delay3 : xbsIndex_r4/Delay  en=off  latency=2  dbl_ovrd=off  reg_retiming=off  block_type=delay
      settings: infoedit=Hardware notes: A delay line is a chain, each link of which is an SRL16 followed by a flip-flop. If register retiming is enabled, the delay line is a chain of flip-flops.  block_version=VER_STRING_GOES_HERE
  Delay4 : xbsIndex_r4/Delay  en=off  latency=1  dbl_ovrd=off  reg_retiming=off  block_type=delay
      settings: infoedit=Hardware notes: A delay line is a chain, each link of which is an SRL16 followed by a flip-flop. If register retiming is enabled, the delay line is a chain of flip-flops.  block_version=VER_STRING_GOES_HERE
  Delay5 : xbsIndex_r4/Delay  en=off  latency=64  dbl_ovrd=off  reg_retiming=off  block_type=delay
      settings: infoedit=Hardware notes: A delay line is a chain, each link of which is an SRL16 followed by a flip-flop. If register retiming is enabled, the delay line is a chain of flip-flops.  block_version=VER_STRING_GOES_HERE
  FFT : Subsystem
  Input Buffers : Subsystem
  Output Buffers : Subsystem
  Rx Buffers Input : Scope  NumInputPorts=6
      settings: TimeRange=6000  YMin=1~-0.2~-0.25~-1~-1~1  YMax=1~0.25~0.2~1~1~1  SaveName=ScopeData46
  Rx Buffers Input1 : Scope  NumInputPorts=6
      settings: TimeRange=4500  YMin=12.35~59.85~-1~-1~-1~-1  YMax=13.65~66.15~1~1~1~1  SaveName=ScopeData55
  Symbol Counter : Subsystem
  done{1,7..9} (4 alike) : xbsIndex_r4/Gateway Out  hdl_port=off  timing_constraint=None  locs_specified=off  LOCs={}  block_type=gatewayout
      settings: infoedit="Gateway out block.  Converts Xilinx fixed point inputs into ouputs of type Simulink integer, double, or fixed point.<P><P>Hardware notes:  In hardware these blocks become top level output ports or are discarded, depending on how they are configured."  xl_area=[0 0 0 0 1 0 0]  block_version=VER_STRING_GOES_HERE
  done{3,6,11..12} (4 alike) : xbsIndex_r4/Gateway Out  Orientation=left  hdl_port=off  timing_constraint=None  locs_specified=off  LOCs={}  block_type=gatewayout
      settings: infoedit="Gateway out block.  Converts Xilinx fixed point inputs into ouputs of type Simulink integer, double, or fixed point.<P><P>Hardware notes:  In hardware these blocks become top level output ports or are discarded, depending on how they are configured."  xl_area=[0 0 0 0 1 0 0]  block_version=VER_STRING_GOES_HERE
  Y_I : Outport
  Y_Q : Outport  Port=2
  H-I : Outport  Port=3
  H-Q : Outport  Port=4
  SubCar Index : Outport  Port=5
  Sym Index : Outport  Port=6
  Vout : Outport  Port=7
  tag scope: FFT_I=global
  wires:
  Addr_Generator/1(Write Addr) -> Input Buffers/4(Wr Addr)
  Input Buffers/1(" I") -> FFT/1(xn_I)
  Input Buffers/2(" Q") -> FFT/2(xn_Q)
  Addr_Generator/5(FFT Start) -> FFT/3(Start)
  Addr_Generator/2(Read Addr) -> Input Buffers/5(Rd Addr)
  FFT/6(xn_Index) -> Assert/1
  Payload/1 -> Addr_Generator/1(Payload), Delay1/1, done8/1, Symbol Counter/2(Payload), Addr Gen/2(Payload)
  AntA I/1 -> Input Buffers/1(AntA I), done9/1
  AntA Q/1 -> Input Buffers/2(AntA Q), done7/1
  Assert/1 -> Addr_Generator/2(FFT Xn_index)
  Addr_Generator/3(Ant Sel) -> Input Buffers/6(Ant Sel)
  FFT/1(xk_I) -> Output Buffers/1(I), Channel Estimation/1(I), [FFT_I]
  FFT/2(xk_Q) -> Output Buffers/2(Q), Channel Estimation/2(Q)
  FFT/3(xk_Index) -> Output Buffers/3(Wr Addr), Channel Estimation/3(Xk Index)
  FFT/4(Vout) -> Output Buffers/4(FFT Vout), Channel Estimation/4(Vin)
  Symbol Counter/1(Sym Index) -> Output Buffers/5(SymIndex), Channel Estimation/5(Sym Index), Delay3/1
  FFT/5(Done) -> Symbol Counter/1(FFT Done), Addr Gen/1(FFT Done), done3/1
  Delay1/1 -> Input Buffers/3(We), done1/1
  done8/1 -> Rx Buffers Input/1
  done9/1 -> Rx Buffers Input/2
  done7/1 -> Rx Buffers Input/3
  done1/1 -> Rx Buffers Input/6
  Addr Gen/1(Rd Addr) -> Output Buffers/6(Rd Addr), Channel Estimation/6(Est Index), Delay4/1
  Channel Estimation/3(Est Vout) -> Addr Gen/3(NotTraining)
  Output Buffers/1(" I") -> Y_I/1
  Channel Estimation/1(H-I) -> H-I/1
  Channel Estimation/2(H-Q) -> H-Q/1
  Output Buffers/2(" Q") -> Y_Q/1
  Addr Gen/2(Vout) -> Delay2/1
  Delay2/1 -> Vout/1, done6/1
  Delay5/1 -> Sym Index/1, done11/1
  Delay4/1 -> SubCar Index/1, done12/1
  done11/1 -> Rx Buffers Input1/1
  done12/1 -> Rx Buffers Input1/2
  done6/1 -> Rx Buffers Input1/3
  done3/1 -> Rx Buffers Input1/4
  Delay3/1 -> Delay5/1
  Addr_Generator/4(Wr Slot) -> Input Buffers/7(Wr Slot)

== system ofdm_txrx_alamouti/OFDM Rx Alamouti/Long Corr 4x ==
  note: changed latency to 0
  note: changed latency to zero
  I In : Inport
  Q In : Inport  Port=2
  AddSub : xbsIndex_r4/AddSub  mode=Subtraction  use_carryin=off  use_carryout=off  en=off  latency=0  precision=Full  arith_type=Unsigned  n_bits=8  bin_pt=2  quantization=Truncate  overflow=Wrap  dbl_ovrd=off  use_behavioral_HDL=on  pipelined=off  use_rpm=off  block_type=addsub
      settings: xl_area=[5 0 0 8 0 0 0]  block_version=VER_STRING_GOES_HERE
  AddSub1 : xbsIndex_r4/AddSub  mode=Addition  use_carryin=off  use_carryout=off  en=off  latency=0  precision=Full  arith_type=Unsigned  n_bits=8  bin_pt=2  quantization=Truncate  overflow=Wrap  dbl_ovrd=off  use_behavioral_HDL=on  pipelined=off  use_rpm=off  block_type=addsub
      settings: xl_area=[5 0 0 8 0 0 0]  block_version=VER_STRING_GOES_HERE
  AddSub2 : xbsIndex_r4/AddSub  mode=Addition  use_carryin=off  use_carryout=off  en=off  latency=0  precision=Full  arith_type=Unsigned  n_bits=8  bin_pt=2  quantization=Truncate  overflow=Wrap  dbl_ovrd=off  use_behavioral_HDL=on  pipelined=off  use_rpm=off  block_type=addsub
      settings: xl_area=[10 0 0 18 0 0 0]  block_version=VER_STRING_GOES_HERE
  C1 : Subsystem  AncestorBlock=ofdm_rx_pom36_cfglib/correlator2/Original Subsystem/C1  mask{ns=0, N=4, h=hr(1:65), Ts_In=4}
      mask prompts: ns "Num sub-correlator"; N "Coefficients/correlator"; h "Coefficients"; Ts_In "Input sample period"
  C2 : Subsystem  AncestorBlock=ofdm_rx_pom36_cfglib/correlator2/Original Subsystem/C1  mask{ns=0, N=4, h=hi(1:65), Ts_In=4}
      mask prompts: ns "Num sub-correlator"; N "Coefficients/correlator"; h "Coefficients"; Ts_In "Input sample period"
  C3 : Subsystem  AncestorBlock=ofdm_rx_pom36_cfglib/correlator2/Original Subsystem/C1  mask{ns=0, N=4, h=hi(1:65), Ts_In=4}
      mask prompts: ns "Num sub-correlator"; N "Coefficients/correlator"; h "Coefficients"; Ts_In "Input sample period"
  C4 : Subsystem  AncestorBlock=ofdm_rx_pom36_cfglib/correlator2/Original Subsystem/C1  mask{ns=0, N=4, h=hr(1:65), Ts_In=4}
      mask prompts: ns "Num sub-correlator"; N "Coefficients/correlator"; h "Coefficients"; Ts_In "Input sample period"
  Gateway Out{3..8} (6 alike) : xbsIndex_r4/Gateway Out  hdl_port=off  timing_constraint=None  locs_specified=off  LOCs={}  block_type=gatewayout
      settings: infoedit="Gateway out block.  Converts Xilinx fixed point inputs into ouputs of type Simulink integer, double, or fixed point.<P><P>Hardware notes:  In hardware these blocks become top level output ports or are discarded, depending on how they are configured."  xl_area=[0 0 0 0 0 0 0]  block_version=VER_STRING_GOES_HERE
  Long Corr Scope4x : Scope  NumInputPorts=6
      settings: YMin=-1~-1~-30~-30~-20~-25  YMax=1~1~70~70~20~20  SaveName=ScopeData2
  Mult : xbsIndex_r4/Mult  precision=Full  arith_type="Signed  (2's comp)"  n_bits=8  bin_pt=2  quantization=Truncate  overflow=Wrap  en=off  latency=1  dbl_ovrd=off  use_behavioral_HDL=off  use_embedded=on  opt=Speed  optimum_pipeline=off  use_rpm=off  placement_style=Rectangular shape  pipeline=on  block_type=mult
      settings: infoedit=Hardware notes: To use the internal pipeline stage of the dedicated multiplier you must select 'Pipeline for maximum performance'.  xl_area=[60 18 0 106 0 0 0]  block_version=VER_STRING_GOES_HERE
  Mult1 : xbsIndex_r4/Mult  precision=Full  arith_type="Signed  (2's comp)"  n_bits=8  bin_pt=2  quantization=Truncate  overflow=Wrap  en=off  latency=1  dbl_ovrd=off  use_behavioral_HDL=off  use_embedded=on  opt=Speed  optimum_pipeline=off  use_rpm=off  placement_style=Rectangular shape  pipeline=on  block_type=mult
      settings: infoedit=Hardware notes: To use the internal pipeline stage of the dedicated multiplier you must select 'Pipeline for maximum performance'.  xl_area=[60 18 0 106 0 0 0]  block_version=VER_STRING_GOES_HERE
  Threshold1 : xbsIndex_r4/Threshold  en=off  latency=0  dbl_ovrd=off  block_type=sgn
      settings: infoedit="Presents -1 as output if input is negative; otherwise presents 1.  Output is a two bit signed number."  xl_area=[0 0 0 0 0 0 0]  block_version=VER_STRING_GOES_HERE
  Threshold2 : xbsIndex_r4/Threshold  en=off  latency=0  dbl_ovrd=off  block_type=sgn
      settings: infoedit="Presents -1 as output if input is negative; otherwise presents 1.  Output is a two bit signed number."  xl_area=[0 0 0 0 0 0 0]  block_version=VER_STRING_GOES_HERE
  y : Outport
  wires:
  C1/1(y) -> AddSub/1, Gateway Out6/1
  AddSub2/1 -> y/1
  Mult1/1 -> AddSub2/2
  Mult/1 -> AddSub2/1
  AddSub1/1 -> Mult1/2, Mult1/1
  AddSub/1 -> Mult/2, Mult/1
  C4/1(y) -> AddSub1/2, Gateway Out5/1
  C3/1(y) -> AddSub1/1, Gateway Out8/1
  C2/1(y) -> AddSub/2, Gateway Out7/1
  Q In/1 -> Threshold1/1, Gateway Out4/1
  I In/1 -> Threshold2/1, Gateway Out3/1
  Threshold2/1 -> C1/1(x), C3/1(x)
  Threshold1/1 -> C2/1(x), C4/1(x)
  Gateway Out3/1 -> Long Corr Scope4x/1
  Gateway Out4/1 -> Long Corr Scope4x/2
  Gateway Out5/1 -> Long Corr Scope4x/6
  Gateway Out6/1 -> Long Corr Scope4x/3
  Gateway Out7/1 -> Long Corr Scope4x/4
  Gateway Out8/1 -> Long Corr Scope4x/5
